simulink model "wlan_phy_rx_pmd"  (Simulink 7.8)
settings: Solver=VariableStepDiscrete  SolverMode=SingleTasking  StartTime=0.0  StopTime=rx_sim.sim_time  RelTol=1e-3
PostLoadFcn:
  | wlan_phy_rx_init
InitFcn:
  | wlan_phy_rx_init
StartFcn:
  | wlan_phy_rx_init
values from neighbouring files (symbols this model uses):
  barker_seq20 = 0.95 * circshift(barker_seq20, [0 4]) ./ max(abs(barker_seq20))    [wlan_phy_rx_init.m]
  bit_scrambler_lfsr_bytes = sum(bit_scrambler_lfsr_bytes .* repmat(2.^[0:7], 127, 1),2)    [wlan_phy_rx_init.m]
  longCorr_coef_i = [3*real(longCorr_coef)]    [wlan_phy_rx_init.m]
  longCorr_coef_q = [3*imag(longCorr_coef)]    [wlan_phy_rx_init.m]
  MAX_CP_LEN = 32    [wlan_phy_rx_init.m]
  MAX_NCBPS = NCBPS_11n_64QAM    (= 312)    [wlan_phy_rx_init.m]
  MAX_NUM_BYTES = 2^16-1    (= 65535)    [wlan_phy_rx_init.m]
  MAX_NUM_SC = 64    [wlan_phy_rx_init.m]
  MAX_NUM_SYMS = 600    [wlan_phy_rx_init.m]
  NDBPS_11a_BPSK12 = 24    [wlan_phy_rx_init.m]
  preFFT_sampBuff_numSamps = 4*MAX_NUM_SC    (= 256)    [wlan_phy_rx_init.m]
  rx_sim = struct()    [wlan_phy_rx_init.m]
  scr_ind_rev = zeros(1,128)    [wlan_phy_rx_init.m]
undefined symbols (not defined in the file or in its folder): gcbh, mcs_rom_11ag, mcs_rom_11n, wlan_rx_deinterleave_rom_gen, xlBlockLoadCallback, xlGetNormalizedPeriod, xlipgetpartsetting
libraries: xbsIndex_r4 x4943, xbsEDKLib_r4 x1
(part 7 of 28)

== system wlan_phy_rx_pmd/Inputs/RF D Inputs ==
  Rst : Inport
  IQ Valid : Inport  Port=2
  Constant1 : Constant  Value=0
  Constant2 : Constant  Value=0
  Constant3 : Constant  Value=0
  Constant6 : Constant  Value=2
  Constant7 : Constant  Value=5
  Delay4 : xbsIndex_r4/Delay  rst=off  infoeditControl=Selection of Reset will increase slice count due to use of real FFs and instead of SRLs  en=off  latency=1  dbl_ovrd=off  reg_retiming=off  block_type=delay
  Delay5 : xbsIndex_r4/Delay  rst=off  infoeditControl=Selection of Reset will increase slice count due to use of real FFs and instead of SRLs  en=off  latency=1  dbl_ovrd=off  reg_retiming=off  block_type=delay
  Down Sample : xbsIndex_r4/Down Sample  sample_ratio=16  sample_phase="Last Value of Frame  (most efficient)"  en=off  latency=1  dbl_ovrd=off  block_type=dsamp
  RF D RSSI Sum : Subsystem
  RFD_G_BB : xbsIndex_r4/Gateway In  gui_display_data_type=Fixed-point  arith_type=Unsigned  n_bits=5  bin_pt=0  preci_type=Single  exp_width=8  frac_width=24  quantization="Round  (unbiased: +/- Inf)"  overflow=Saturate  period=1  dbl_ovrd=off  timing_constraint=None  locs_specified=off  LOCs={}  UseAsADC=off  ADCChannel='1'  inherit_from_input=off  hdl_port=on  block_type=gatewayin
  RFD_G_RF : xbsIndex_r4/Gateway In  gui_display_data_type=Fixed-point  arith_type=Unsigned  n_bits=2  bin_pt=0  preci_type=Single  exp_width=8  frac_width=24  quantization="Round  (unbiased: +/- Inf)"  overflow=Saturate  period=1  dbl_ovrd=off  timing_constraint=None  locs_specified=off  LOCs={}  UseAsADC=off  ADCChannel='1'  inherit_from_input=off  hdl_port=on  block_type=gatewayin
  RFD_RSSI : xbsIndex_r4/Gateway In  gui_display_data_type=Fixed-point  arith_type=Unsigned  n_bits=10  bin_pt=0  preci_type=Single  exp_width=8  frac_width=24  quantization="Round  (unbiased: +/- Inf)"  overflow=Saturate  period=1  dbl_ovrd=off  timing_constraint=None  locs_specified=off  LOCs={}  UseAsADC=off  ADCChannel='1'  inherit_from_input=off  hdl_port=on  block_type=gatewayin
  RFD_RX_I : xbsIndex_r4/Gateway In  gui_display_data_type=Fixed-point  arith_type="Signed  (2's comp)"  n_bits=16  bin_pt=15  preci_type=Single  exp_width=8  frac_width=24  quantization="Round  (unbiased: +/- Inf)"  overflow=Saturate  period=1  dbl_ovrd=off  timing_constraint=None  locs_specified=off  LOCs={}  UseAsADC=off  ADCChannel='1'  inherit_from_input=off  hdl_port=on  block_type=gatewayin
  RFD_RX_Q : xbsIndex_r4/Gateway In  gui_display_data_type=Fixed-point  arith_type="Signed  (2's comp)"  n_bits=16  bin_pt=15  preci_type=Single  exp_width=8  frac_width=24  quantization="Round  (unbiased: +/- Inf)"  overflow=Saturate  period=1  dbl_ovrd=off  timing_constraint=None  locs_specified=off  LOCs={}  UseAsADC=off  ADCChannel='1'  inherit_from_input=off  hdl_port=on  block_type=gatewayin
  Register : xbsIndex_r4/Register  init=0  rst=on  en=on  dbl_ovrd=off  block_type=register
  Register1 : xbsIndex_r4/Register  init=0  rst=on  en=on  dbl_ovrd=off  block_type=register
  Register2 : xbsIndex_r4/Register  init=0  rst=off  en=off  dbl_ovrd=off  block_type=register
  Register3 : xbsIndex_r4/Register  init=0  rst=on  en=off  dbl_ovrd=off  block_type=register
  Register4 : xbsIndex_r4/Register  init=0  rst=off  en=off  dbl_ovrd=off  block_type=register
  Register5 : xbsIndex_r4/Register  init=0  rst=off  en=off  dbl_ovrd=off  block_type=register
  ADC I : Outport
  ADC Q : Outport  Port=2
  Avg RSSI : Outport  Port=3
  tag scope: RFD_AGC_G_BB=global, RFD_AGC_G_RF=global
  wires:
  Constant7/1 -> RFD_G_BB/1
  Constant6/1 -> RFD_G_RF/1
  Delay5/1 -> [RFD_AGC_G_BB]
  Delay4/1 -> [RFD_AGC_G_RF]
  RFD_G_BB/1 -> Delay5/1
  RFD_G_RF/1 -> Delay4/1
  RFD_RX_I/1 -> Register2/1
  RFD_RX_Q/1 -> Register4/1
  Register/1 -> ADC I/1
  Register1/1 -> ADC Q/1
  Register3/1 -> Down Sample/1
  Down Sample/1 -> RF D RSSI Sum/1(RSSI)
  RF D RSSI Sum/1(Sum) -> Avg RSSI/1
  RFD_RSSI/1 -> Register5/1
  Register2/1 -> Register/1
  Register4/1 -> Register1/1
  Register5/1 -> Register3/1
  Rst/1 -> Register/2, Register1/2, Register3/2
  IQ Valid/1 -> Register1/3, Register/3
  Constant1/1 -> RFD_RX_I/1
  Constant2/1 -> RFD_RX_Q/1
  Constant3/1 -> RFD_RSSI/1

== system wlan_phy_rx_pmd/Inputs/RSSI Clock Gen ==
  1LSB+3 : xbsIndex_r4/Slice  nbits=1  boolean_output=off  mode=Lower Bit Location + Width  bit1=0  base1=MSB of Input  bit0=3  base0=LSB of Input  dbl_ovrd=off  block_type=slice
  RSSI Clock Generator : xbsIndex_r4/Counter  cnt_type=Free Running  cnt_to=Inf  operation=Up  start_count=0  cnt_by_val=1  arith_type=Unsigned  n_bits=4  bin_pt=0  load_pin=off  rst=off  en=off  explicit_period=on  period=1  dbl_ovrd=off  use_behavioral_HDL=off  implementation=Fabric  use_rpm=off  block_type=counter
      settings: block_version=VER_STRING_GOES_HERE
  Clk : Outport
  wires:
  RSSI Clock Generator/1 -> 1LSB+3/1
  1LSB+3/1 -> Clk/1

== system wlan_phy_rx_pmd/Inputs/negedge1 ==
  D : Inport
  Delay : xbsIndex_r4/Delay  rst=off  infoeditControl=Selection of Reset will increase slice count due to use of real FFs and instead of SRLs  en=off  latency=1  dbl_ovrd=off  reg_retiming=off  block_type=delay
      settings: infoedit=Hardware notes: A delay line is a chain, each link of which is an SRL16 followed by a flip-flop. If register retiming is enabled, the delay line is a chain of flip-flops.  xl_area=[1 1 0 0 0 0 0]  block_version=VER_STRING_GOES_HERE
  Inverter : xbsIndex_r4/Inverter  en=off  latency=0  dbl_ovrd=off  block_type=inv
      settings: xl_area=[0 0 0 0 0 0 0]  block_version=VER_STRING_GOES_HERE
  Logical1 : xbsIndex_r4/Logical  logical_function=AND  inputs=2  en=off  latency=0  precision=Full  arith_type=Unsigned  n_bits=8  bin_pt=2  align_bp=on  dbl_ovrd=off  block_type=logical
      settings: xl_area=[1 0 0 1 0 0 0]  block_version=VER_STRING_GOES_HERE
  Q : Outport
  wires:
  D/1 -> Delay/1, Inverter/1
  Logical1/1 -> Q/1
  Delay/1 -> Logical1/1
  Inverter/1 -> Logical1/2

== system wlan_phy_rx_pmd/MAC I/O/Pulse Stretch1 ==
  P : Inport
  Delay : xbsIndex_r4/Delay  rst=off  infoeditControl=Selection of Reset will increase slice count due to use of real FFs and instead of SRLs  en=off  latency=16  dbl_ovrd=off  reg_retiming=off  block_type=delay
  Register15 : xbsIndex_r4/Register  init=0  rst=off  en=off  dbl_ovrd=off  block_type=register
  S-R Latch : Subsystem
  P16 : Outport
  wires:
  S-R Latch/1(Q) -> Delay/1, Register15/1
  Delay/1 -> S-R Latch/2(R)
  P/1 -> S-R Latch/1(S)
  Register15/1 -> P16/1

== system wlan_phy_rx_pmd/MAC I/O/S-R Latch1 ==
  S : Inport
  R : Inport  Port=2
  Constant1 : xbsIndex_r4/Constant  const=1  gui_display_data_type=Fixed-point  arith_type=Boolean  n_bits=1  bin_pt=0  preci_type=Single  exp_width=8  frac_width=24  explicit_period=off  period=1  equ=P=C  opselect=C  inp2=PCIN>>17  opr=+  inp1=P  carry=CIN  dbl_ovrd=off  block_type=constant
      settings: block_version=VER_STRING_GOES_HERE
  Convert1 : xbsIndex_r4/Convert  gui_display_data_type=Boolean  arith_type=Boolean  n_bits=1  bin_pt=0  float_type=Single  exp_bits=8  fraction_bits=24  quantization=Truncate  overflow=Wrap  en=off  latency=0  dbl_ovrd=off  pipeline=off  block_type=convert
  Convert2 : xbsIndex_r4/Convert  gui_display_data_type=Boolean  arith_type=Boolean  n_bits=1  bin_pt=0  float_type=Single  exp_bits=8  fraction_bits=24  quantization=Truncate  overflow=Wrap  en=off  latency=0  dbl_ovrd=off  pipeline=off  block_type=convert
  Register2 : xbsIndex_r4/Register  init=0  rst=on  en=on  dbl_ovrd=off  block_type=register
      settings: xl_area=[1 1 0 0 0 0 0]  block_version=VER_STRING_GOES_HERE
  Q : Outport
  wires:
  Convert2/1 -> Register2/3
  Convert1/1 -> Register2/2
  Constant1/1 -> Register2/1
  R/1 -> Convert1/1
  Register2/1 -> Q/1
  S/1 -> Convert2/1

== system wlan_phy_rx_pmd/MAC I/O/S-R Latch2 ==
  S : Inport
  R : Inport  Port=2
  Constant1 : xbsIndex_r4/Constant  const=1  gui_display_data_type=Fixed-point  arith_type=Boolean  n_bits=1  bin_pt=0  preci_type=Single  exp_width=8  frac_width=24  explicit_period=off  period=1  equ=P=C  opselect=C  inp2=PCIN>>17  opr=+  inp1=P  carry=CIN  dbl_ovrd=off  block_type=constant
      settings: block_version=VER_STRING_GOES_HERE
  Convert1 : xbsIndex_r4/Convert  gui_display_data_type=Boolean  arith_type=Boolean  n_bits=1  bin_pt=0  float_type=Single  exp_bits=8  fraction_bits=24  quantization=Truncate  overflow=Wrap  en=off  latency=0  dbl_ovrd=off  pipeline=off  block_type=convert
  Convert2 : xbsIndex_r4/Convert  gui_display_data_type=Boolean  arith_type=Boolean  n_bits=1  bin_pt=0  float_type=Single  exp_bits=8  fraction_bits=24  quantization=Truncate  overflow=Wrap  en=off  latency=0  dbl_ovrd=off  pipeline=off  block_type=convert
  Register2 : xbsIndex_r4/Register  init=0  rst=on  en=on  dbl_ovrd=off  block_type=register
      settings: xl_area=[1 1 0 0 0 0 0]  block_version=VER_STRING_GOES_HERE
  Q : Outport
  wires:
  S/1 -> Convert2/1
  Register2/1 -> Q/1
  R/1 -> Convert1/1
  Constant1/1 -> Register2/1
  Convert1/1 -> Register2/2
  Convert2/1 -> Register2/3

== system wlan_phy_rx_pmd/MAC I/O/posedge ==
  D : Inport
  Delay : xbsIndex_r4/Delay  rst=off  infoeditControl=Selection of Reset will increase slice count due to use of real FFs and instead of SRLs  en=off  latency=1  dbl_ovrd=off  reg_retiming=off  block_type=delay
      settings: infoedit=Hardware notes: A delay line is a chain, each link of which is an SRL16 followed by a flip-flop. If register retiming is enabled, the delay line is a chain of flip-flops.  xl_area=[1 1 0 0 0 0 0]  block_version=VER_STRING_GOES_HERE
  Inverter : xbsIndex_r4/Inverter  en=off  latency=0  dbl_ovrd=off  block_type=inv
      settings: xl_area=[0 0 0 0 0 0 0]  block_version=VER_STRING_GOES_HERE
  Logical1 : xbsIndex_r4/Logical  logical_function=AND  inputs=2  en=off  latency=0  precision=Full  arith_type=Unsigned  n_bits=8  bin_pt=2  align_bp=on  dbl_ovrd=off  block_type=logical
      settings: xl_area=[1 0 0 1 0 0 0]  block_version=VER_STRING_GOES_HERE
  Q : Outport
  wires:
  Inverter/1 -> Logical1/2
  Logical1/1 -> Q/1
  D/1 -> Delay/1, Logical1/1
  Delay/1 -> Inverter/1

== system wlan_phy_rx_pmd/Registers/2x  ==
  d : Inport
  Concat2 : xbsIndex_r4/Concat  num_inputs=2  dbl_ovrd=off  block_type=concat
      settings: block_version=8.2.02
  Constant2 : xbsIndex_r4/Constant  const=0  gui_display_data_type=Fixed-point  arith_type=Unsigned  n_bits=1  bin_pt=0  preci_type=Single  exp_width=8  frac_width=24  explicit_period=on  period=1  equ=P=C  opselect=C  inp2=PCIN>>17  opr=+  inp1=P  carry=CIN  dbl_ovrd=off  block_type=constant
  2d : Outport
  wires:
  Concat2/1 -> 2d/1
  d/1 -> Concat2/1
  Constant2/1 -> Concat2/2

== system wlan_phy_rx_pmd/Registers/Config Bits ==
  32b : Inport
  "b[0]   " : xbsIndex_r4/Slice  nbits=1  boolean_output=on  mode=Lower Bit Location + Width  bit1=0  base1=MSB of Input  bit0=0  base0=LSB of Input  dbl_ovrd=off  block_type=slice
      settings: block_version=VER_STRING_GOES_HERE
  b[10] : xbsIndex_r4/Slice  nbits=1  boolean_output=on  mode=Lower Bit Location + Width  bit1=0  base1=MSB of Input  bit0=10  base0=LSB of Input  dbl_ovrd=off  block_type=slice
      settings: block_version=VER_STRING_GOES_HERE
  b[11] : xbsIndex_r4/Slice  nbits=1  boolean_output=on  mode=Lower Bit Location + Width  bit1=0  base1=MSB of Input  bit0=11  base0=LSB of Input  dbl_ovrd=off  block_type=slice
      settings: block_version=VER_STRING_GOES_HERE
  b[12] : xbsIndex_r4/Slice  nbits=1  boolean_output=on  mode=Lower Bit Location + Width  bit1=0  base1=MSB of Input  bit0=12  base0=LSB of Input  dbl_ovrd=off  block_type=slice
      settings: block_version=VER_STRING_GOES_HERE
  b[13] : xbsIndex_r4/Slice  nbits=1  boolean_output=on  mode=Lower Bit Location + Width  bit1=0  base1=MSB of Input  bit0=13  base0=LSB of Input  dbl_ovrd=off  block_type=slice
      settings: block_version=VER_STRING_GOES_HERE
  b[14] : xbsIndex_r4/Slice  nbits=1  boolean_output=on  mode=Lower Bit Location + Width  bit1=0  base1=MSB of Input  bit0=14  base0=LSB of Input  dbl_ovrd=off  block_type=slice
      settings: block_version=VER_STRING_GOES_HERE
  b[16:15] : xbsIndex_r4/Slice  nbits=2  boolean_output=off  mode=Lower Bit Location + Width  bit1=0  base1=MSB of Input  bit0=15  base0=LSB of Input  dbl_ovrd=off  block_type=slice
      settings: block_version=VER_STRING_GOES_HERE
  "b[1]  " : xbsIndex_r4/Slice  nbits=1  boolean_output=on  mode=Lower Bit Location + Width  bit1=0  base1=MSB of Input  bit0=1  base0=LSB of Input  dbl_ovrd=off  block_type=slice
      settings: block_version=VER_STRING_GOES_HERE
  b[20:17] : xbsIndex_r4/Slice  nbits=4  boolean_output=off  mode=Lower Bit Location + Width  bit1=0  base1=MSB of Input  bit0=17  base0=LSB of Input  dbl_ovrd=off  block_type=slice
      settings: block_version=VER_STRING_GOES_HERE
  b[21] : xbsIndex_r4/Slice  nbits=1  boolean_output=on  mode=Lower Bit Location + Width  bit1=0  base1=MSB of Input  bit0=21  base0=LSB of Input  dbl_ovrd=off  block_type=slice
      settings: block_version=VER_STRING_GOES_HERE
  b[22] : xbsIndex_r4/Slice  nbits=1  boolean_output=on  mode=Lower Bit Location + Width  bit1=0  base1=MSB of Input  bit0=22  base0=LSB of Input  dbl_ovrd=off  block_type=slice
      settings: block_version=VER_STRING_GOES_HERE
  b[23] : xbsIndex_r4/Slice  nbits=1  boolean_output=on  mode=Lower Bit Location + Width  bit1=0  base1=MSB of Input  bit0=23  base0=LSB of Input  dbl_ovrd=off  block_type=slice
      settings: block_version=VER_STRING_GOES_HERE
  b[24] : xbsIndex_r4/Slice  nbits=1  boolean_output=on  mode=Lower Bit Location + Width  bit1=0  base1=MSB of Input  bit0=24  base0=LSB of Input  dbl_ovrd=off  block_type=slice
      settings: block_version=VER_STRING_GOES_HERE
  b[2] : xbsIndex_r4/Slice  nbits=1  boolean_output=off  mode=Lower Bit Location + Width  bit1=0  base1=MSB of Input  bit0=2  base0=LSB of Input  dbl_ovrd=off  block_type=slice
      settings: block_version=VER_STRING_GOES_HERE
  b[3] : xbsIndex_r4/Slice  nbits=1  boolean_output=off  mode=Lower Bit Location + Width  bit1=0  base1=MSB of Input  bit0=3  base0=LSB of Input  dbl_ovrd=off  block_type=slice
      settings: block_version=VER_STRING_GOES_HERE
  b[4] : xbsIndex_r4/Slice  nbits=1  boolean_output=off  mode=Lower Bit Location + Width  bit1=0  base1=MSB of Input  bit0=4  base0=LSB of Input  dbl_ovrd=off  block_type=slice
      settings: block_version=VER_STRING_GOES_HERE
  b[5] : xbsIndex_r4/Slice  nbits=1  boolean_output=on  mode=Lower Bit Location + Width  bit1=0  base1=MSB of Input  bit0=5  base0=LSB of Input  dbl_ovrd=off  block_type=slice
      settings: block_version=VER_STRING_GOES_HERE
  b[6] : xbsIndex_r4/Slice  nbits=1  boolean_output=on  mode=Lower Bit Location + Width  bit1=0  base1=MSB of Input  bit0=6  base0=LSB of Input  dbl_ovrd=off  block_type=slice
      settings: block_version=VER_STRING_GOES_HERE
  b[7] : xbsIndex_r4/Slice  nbits=1  boolean_output=on  mode=Lower Bit Location + Width  bit1=0  base1=MSB of Input  bit0=7  base0=LSB of Input  dbl_ovrd=off  block_type=slice
      settings: block_version=VER_STRING_GOES_HERE
  b[8] : xbsIndex_r4/Slice  nbits=1  boolean_output=on  mode=Lower Bit Location + Width  bit1=0  base1=MSB of Input  bit0=8  base0=LSB of Input  dbl_ovrd=off  block_type=slice
      settings: block_version=VER_STRING_GOES_HERE
  b[9] : xbsIndex_r4/Slice  nbits=1  boolean_output=on  mode=Lower Bit Location + Width  bit1=0  base1=MSB of Input  bit0=9  base0=LSB of Input  dbl_ovrd=off  block_type=slice
      settings: block_version=VER_STRING_GOES_HERE
  tag scope: REG_USE_RX_SIGS_INVALID_INPUT=global, regRx_BYPASS_CFO_EST=global, regRx_DSSS_RX_EN=global, regRx_DSSS_RX_PKT_DET_OUT_EN=global, regRx_DSSS_RX_REQ_AGC_DONE=global, regRx_DSSS_asserts_CCA_busy=global, regRx_MAX_SIGNAL_LENGTH_KB=global, regRx_PHY_MODE_11N_ENABLE=global, regRx_PKT_BUF_BYTE_ORDER_SWAP=global, regRx_PKT_BUF_H_EST_ORDER_SWAP=global, regRx_Record_Chan_Est_Pkt_Buf=global, regRx_noSwitching_AntSel=global, regRx_phyCCA_Mode_Sel=global, regRx_pktDetEn_Ext=global, regRx_pktDetEn_RFA=global, regRx_pktDetEn_RFB=global, regRx_pktDetEn_RFC=global, regRx_pktDetEn_RFD=global, regRx_pktDet_requireBoth=global, regRx_rateLength_holds_pktDet=global, regRx_switchingDiv_En=global
  wires:
  32b/1 -> "b[1]  "/1, b[2]/1, b[3]/1, b[4]/1, b[5]/1, b[6]/1, b[7]/1, b[9]/1, b[10]/1, b[12]/1, b[13]/1, b[14]/1, b[16:15]/1, b[20:17]/1, b[21]/1, b[22]/1, b[23]/1, b[24]/1, b[11]/1, b[8]/1, "b[0]   "/1
  "b[0]   "/1 -> [regRx_DSSS_RX_EN]
  "b[1]  "/1 -> [REG_USE_RX_SIGS_INVALID_INPUT]
  b[2]/1 -> [regRx_PKT_BUF_BYTE_ORDER_SWAP]
  b[3]/1 -> [regRx_PKT_BUF_H_EST_ORDER_SWAP]
  b[4]/1 -> [regRx_DSSS_RX_PKT_DET_OUT_EN]
  b[5]/1 -> [regRx_BYPASS_CFO_EST]
  b[6]/1 -> [regRx_Record_Chan_Est_Pkt_Buf]
  b[7]/1 -> [regRx_switchingDiv_En]
  b[16:15]/1 -> [regRx_noSwitching_AntSel]
  b[9]/1 -> [regRx_pktDetEn_RFA]
  b[10]/1 -> [regRx_pktDetEn_RFB]
  b[13]/1 -> [regRx_pktDetEn_Ext]
  b[11]/1 -> [regRx_pktDetEn_RFC]
  b[12]/1 -> [regRx_pktDetEn_RFD]
  b[14]/1 -> [regRx_phyCCA_Mode_Sel]
  b[8]/1 -> [regRx_DSSS_RX_REQ_AGC_DONE]
  b[20:17]/1 -> [regRx_MAX_SIGNAL_LENGTH_KB]
  b[21]/1 -> [regRx_pktDet_requireBoth]
  b[22]/1 -> [regRx_rateLength_holds_pktDet]
  b[23]/1 -> [regRx_DSSS_asserts_CCA_busy]
  b[24]/1 -> [regRx_PHY_MODE_11N_ENABLE]

== system wlan_phy_rx_pmd/Registers/Fanout ==
  Corr_Thresh : Inport
  E_Thresh : Inport  Port=2
  MinDur : Inport  Port=3
  Register{1..11,20} (12 alike) : xbsIndex_r4/Register  init=0  rst=off  en=off  dbl_ovrd=off  block_type=register
  tag scope: regPktDet_pktDetMinDuration_autoCorr_A=global, regPktDet_pktDetMinDuration_autoCorr_B=global, regPktDet_pktDetMinDuration_autoCorr_C=global, regPktDet_pktDetMinDuration_autoCorr_D=global, regRx_PktDet_CorrThresh_A=global, regRx_PktDet_CorrThresh_B=global, regRx_PktDet_CorrThresh_C=global, regRx_PktDet_CorrThresh_D=global, regRx_PktDet_EnergyThresh_A=global, regRx_PktDet_EnergyThresh_B=global, regRx_PktDet_EnergyThresh_C=global, regRx_PktDet_EnergyThresh_D=global
  wires:
  Corr_Thresh/1 -> Register20/1, Register1/1, Register2/1, Register3/1
  E_Thresh/1 -> Register4/1, Register5/1, Register6/1, Register7/1
  MinDur/1 -> Register8/1, Register9/1, Register10/1, Register11/1
  Register20/1 -> [regRx_PktDet_CorrThresh_D]
  Register1/1 -> [regRx_PktDet_CorrThresh_C]
  Register2/1 -> [regRx_PktDet_CorrThresh_B]
  Register3/1 -> [regRx_PktDet_CorrThresh_A]
  Register4/1 -> [regRx_PktDet_EnergyThresh_A]
  Register5/1 -> [regRx_PktDet_EnergyThresh_B]
  Register6/1 -> [regRx_PktDet_EnergyThresh_C]
  Register7/1 -> [regRx_PktDet_EnergyThresh_D]
  Register8/1 -> [regPktDet_pktDetMinDuration_autoCorr_A]
  Register9/1 -> [regPktDet_pktDetMinDuration_autoCorr_B]
  Register10/1 -> [regPktDet_pktDetMinDuration_autoCorr_C]
  Register11/1 -> [regPktDet_pktDetMinDuration_autoCorr_D]

== system wlan_phy_rx_pmd/Registers/Fanout1 ==
  In1 : Inport
  In2 : Inport  Port=2
  In3 : Inport  Port=3
  Register{1..11,20} (12 alike) : xbsIndex_r4/Register  init=0  rst=off  en=off  dbl_ovrd=off  block_type=register
  tag scope: regRx_PKTDET_RSSI_MIN_DUR_A=global, regRx_PKTDET_RSSI_MIN_DUR_B=global, regRx_PKTDET_RSSI_MIN_DUR_C=global, regRx_PKTDET_RSSI_MIN_DUR_D=global, regRx_PKTDET_RSSI_THRESH_A=global, regRx_PKTDET_RSSI_THRESH_B=global, regRx_PKTDET_RSSI_THRESH_C=global, regRx_PKTDET_RSSI_THRESH_D=global, regRx_RSSI_SUM_LEN_A=global, regRx_RSSI_SUM_LEN_B=global, regRx_RSSI_SUM_LEN_C=global, regRx_RSSI_SUM_LEN_D=global
  wires:
  Register3/1 -> [regRx_RSSI_SUM_LEN_A]
  Register2/1 -> [regRx_RSSI_SUM_LEN_B]
  Register1/1 -> [regRx_RSSI_SUM_LEN_C]
  Register20/1 -> [regRx_RSSI_SUM_LEN_D]
  In1/1 -> Register3/1, Register2/1, Register1/1, Register20/1
  In2/1 -> Register6/1, Register4/1, Register5/1, Register7/1
  In3/1 -> Register11/1, Register9/1, Register8/1, Register10/1
  Register11/1 -> [regRx_PKTDET_RSSI_MIN_DUR_A]
  Register9/1 -> [regRx_PKTDET_RSSI_MIN_DUR_B]
  Register8/1 -> [regRx_PKTDET_RSSI_MIN_DUR_C]
  Register10/1 -> [regRx_PKTDET_RSSI_MIN_DUR_D]
  Register7/1 -> [regRx_PKTDET_RSSI_THRESH_A]
  Register5/1 -> [regRx_PKTDET_RSSI_THRESH_B]
  Register4/1 -> [regRx_PKTDET_RSSI_THRESH_C]
  Register6/1 -> [regRx_PKTDET_RSSI_THRESH_D]

== system wlan_phy_rx_pmd/Registers/Fanout2 ==
  Corr_Thresh : Inport
  E_Thresh : Inport  Port=2
  Register{1..7,20} (8 alike) : xbsIndex_r4/Register  init=0  rst=off  en=off  dbl_ovrd=off  block_type=register
  tag scope: regRx_PktDet_CorrThresh_DSSS_A=global, regRx_PktDet_CorrThresh_DSSS_B=global, regRx_PktDet_CorrThresh_DSSS_C=global, regRx_PktDet_CorrThresh_DSSS_D=global, regRx_PktDet_EnergyThresh_DSSS_A=global, regRx_PktDet_EnergyThresh_DSSS_B=global, regRx_PktDet_EnergyThresh_DSSS_C=global, regRx_PktDet_EnergyThresh_DSSS_D=global
  wires:
  Register3/1 -> [regRx_PktDet_CorrThresh_DSSS_A]
  Register2/1 -> [regRx_PktDet_CorrThresh_DSSS_B]
  Register1/1 -> [regRx_PktDet_CorrThresh_DSSS_C]
  Register20/1 -> [regRx_PktDet_CorrThresh_DSSS_D]
  Corr_Thresh/1 -> Register3/1, Register2/1, Register1/1, Register20/1
  E_Thresh/1 -> Register4/1, Register5/1, Register6/1, Register7/1
  Register4/1 -> [regRx_PktDet_EnergyThresh_DSSS_A]
  Register5/1 -> [regRx_PktDet_EnergyThresh_DSSS_B]
  Register6/1 -> [regRx_PktDet_EnergyThresh_DSSS_C]
  Register7/1 -> [regRx_PktDet_EnergyThresh_DSSS_D]

== system wlan_phy_rx_pmd/Registers/Sim Reset ==
  Add : Sum
  Disregard Subsystem : xbsIndex_r4/Disregard Subsystem  Tag=discardX  block_type=disregard
  Pulse Generator : DiscretePulseGenerator  Period=1e6  PulseWidth=2  PhaseDelay=1.25e4
  Pulse Generator1 : DiscretePulseGenerator  Period=1e6  PulseWidth=2  PhaseDelay=2.5e4
  sim_only_reset : xbsIndex_r4/Gateway In  gui_display_data_type=Fixed-point  arith_type=Unsigned  n_bits=1  bin_pt=0  preci_type=Single  exp_width=8  frac_width=24  quantization="Round  (unbiased: +/- Inf)"  overflow=Saturate  period=1  dbl_ovrd=off  timing_constraint=None  locs_specified=off  LOCs={}  UseAsADC=off  ADCChannel='1'  inherit_from_input=off  hdl_port=on  block_type=gatewayin
  Out1 : Outport
  wires:
  sim_only_reset/1 -> Out1/1
  Pulse Generator/1 -> Add/1
  Pulse Generator1/1 -> Add/2
  Add/1 -> sim_only_reset/1

== system wlan_phy_rx_pmd/Sync & Antenna Sel/Antenna Selection ==
  Pkt Det : Inport
  Rx I Vec : Inport  Port=2
  Rx Q Vec : Inport  Port=3
  IQ Valid : Inport  Port=4
  RSSI Vec : Inport  Port=5
  Reset : Inport  Port=6
  Ant Sel Logic : Subsystem
  Bus Selector1 : BusSelector  OutputSignals=RFA_Q,RFB_Q,RFC_Q,RFD_Q
  Bus Selector2 : BusSelector  OutputSignals=RFA_RSSI,RFB_RSSI,RFC_RSSI,RFD_RSSI
  Bus Selector3 : BusSelector  OutputSignals=RFA_I,RFB_I,RFC_I,RFD_I
  Bus Selector4 : BusSelector  OutputSignals=signal1,signal2,signal3,signal4
  Delay : xbsIndex_r4/Delay  rst=off  infoeditControl=Selection of Reset will increase slice count due to use of real FFs and instead of SRLs  en=off  latency=1  dbl_ovrd=off  reg_retiming=off  block_type=delay
  Gateway Out{,1..2,4..6} (6 alike) : xbsIndex_r4/Gateway Out  inherit_from_input=off  hdl_port=off  timing_constraint=None  locs_specified=off  LOCs={}  UseAsDAC=off  DACChannel='1'  block_type=gatewayout
  Logical : xbsIndex_r4/Logical  logical_function=AND  inputs=2  en=off  latency=0  precision=Full  arith_type=Unsigned  n_bits=16  bin_pt=0  align_bp=on  dbl_ovrd=off  block_type=logical
  Mux{1..4} (4 alike) : xbsIndex_r4/Mux  inputs=4  en=off  latency=0  precision=Full  arith_type=Unsigned  n_bits=16  bin_pt=14  quantization=Truncate  overflow=Wrap  dbl_ovrd=off  block_type=mux
  Posedge : Subsystem
  RF A Gains : Subsystem
  RF B Gains : Subsystem
  RF C Gains : Subsystem
  RF D Gains : Subsystem
  RSSI & Gain Capture : Subsystem
  Register3 : xbsIndex_r4/Register  init=0  rst=off  en=on  dbl_ovrd=off  block_type=register
      settings: xl_area=[1 1 0 0 0 0 0]  block_version=VER_STRING_GOES_HERE
  Sel Div : Scope  NumInputPorts=10
      settings: TimeRange=16708  YMin=0~-1~-1~0~-1~-1~-1~-1~-1~-1  YMax=1~1~1~1~1~1~1~1~1~1  SaveName=ScopeData42
  Rx I : Outport
  " Rx Q" : Outport  Port=2
  " IQ Valid" : Outport  Port=3
  RSSI : Outport  Port=4
  tag scope: ANTENNA_SEL=global
  wires:
  RF A Gains/1(G_vec) -> Gateway Out2/1, Ant Sel Logic/1(G A)
  RF B Gains/1(G_vec) -> Gateway Out4/1, Ant Sel Logic/2(G B)
  Rx Q Vec/1 -> Bus Selector1/1
  Reset/1 -> RF B Gains/2(Rst), RF A Gains/2(Rst), RF C Gains/2(Rst), RF D Gains/2(Rst)
  Logical/1 -> Posedge/1(D)
  Pkt Det/1 -> Logical/1, Gateway Out/1
  [AGC_DONE] -> Logical/2, Gateway Out1/1
  Posedge/1(Q) -> RF A Gains/1(Capt), RF B Gains/1(Capt), RF C Gains/1(Capt), Delay/1, RF D Gains/1(Capt)
  Delay/1 -> Register3/2
  Register3/1 -> [ANTENNA_SEL]
  IQ Valid/1 -> Bus Selector4/1
  Bus Selector4/1 -> Mux3/2  "<signal1>"
  Bus Selector4/2 -> Mux3/3  "<signal2>"
  Mux3/1 -> " IQ Valid"/1
  Gateway Out2/1 -> Sel Div/3  "G A"
  Gateway Out1/1 -> Sel Div/2  "AGC Done"
  Gateway Out/1 -> Sel Div/1  "Pkt Det"
  Gateway Out4/1 -> Sel Div/4  "G B"
  RF C Gains/1(G_vec) -> Ant Sel Logic/3(G C), Gateway Out5/1
  RF D Gains/1(G_vec) -> Ant Sel Logic/4(G D), Gateway Out6/1
  Gateway Out5/1 -> Sel Div/5  "G C"
  Gateway Out6/1 -> Sel Div/6  "G D"
  Bus Selector4/3 -> Mux3/4  "<signal3>"
  Bus Selector4/4 -> Mux3/5  "<signal4>"
  Rx I Vec/1 -> Bus Selector3/1
  Bus Selector3/1 -> Mux1/2  "<RFA_I>"
  Bus Selector3/2 -> Mux1/3  "<RFB_I>"
  Bus Selector3/3 -> Mux1/4  "<RFC_I>"
  Bus Selector3/4 -> Mux1/5  "<RFD_I>"
  Bus Selector1/1 -> Mux2/2  "<RFA_Q>"
  Bus Selector1/2 -> Mux2/3  "<RFB_Q>"
  Bus Selector1/3 -> Mux2/4  "<RFC_Q>"
  Bus Selector1/4 -> Mux2/5  "<RFD_Q>"
  RSSI Vec/1 -> Bus Selector2/1, RSSI & Gain Capture/1(RSSI Vec)
  Bus Selector2/1 -> Mux4/2  "<RFA_RSSI>"
  Bus Selector2/2 -> Mux4/3  "<RFB_RSSI>"
  Bus Selector2/3 -> Mux4/4  "<RFC_RSSI>"
  Bus Selector2/4 -> Mux4/5  "<RFD_RSSI>"
  Mux4/1 -> RSSI/1
  Ant Sel Logic/1(Ant Sel) -> Mux3/1, Mux1/1, Mux2/1, Mux4/1, Register3/1
  Mux2/1 -> " Rx Q"/1
  Mux1/1 -> Rx I/1

== system wlan_phy_rx_pmd/Sync & Antenna Sel/Debug  Outputs ==
  note: Pulse stretch the timeout signal
      | so it's visible on debug outputs
      | and in down-sampled CS ILA
  LTS : Inport
  LTS Timeout : Inport  Port=2
  Det OFDM : Inport  Port=3
  Det DSSS : Inport  Port=4
  LTS_SYNC : xbsIndex_r4/Gateway Out  inherit_from_input=off  hdl_port=on  timing_constraint=None  locs_specified=off  LOCs={}  UseAsDAC=off  DACChannel='1'  block_type=gatewayout
  Pulse Stretch : Subsystem
  Pulse Stretch1 : Subsystem
  Register{2..11} (10 alike) : xbsIndex_r4/Register  init=0  rst=off  en=off  dbl_ovrd=off  block_type=register
  Slice2 : xbsIndex_r4/Slice  nbits=1  boolean_output=on  mode=Upper Bit Location + Width  bit1=0  base1=MSB of Input  bit0=0  base0=LSB of Input  dbl_ovrd=off  block_type=slice
  dbg_PKT_DET_DSSS : xbsIndex_r4/Gateway Out  inherit_from_input=off  hdl_port=on  timing_constraint=None  locs_specified=off  LOCs={}  UseAsDAC=off  DACChannel='1'  block_type=gatewayout
  dbg_PKT_DET_OFDM : xbsIndex_r4/Gateway Out  inherit_from_input=off  hdl_port=on  timing_constraint=None  locs_specified=off  LOCs={}  UseAsDAC=off  DACChannel='1'  block_type=gatewayout
  dbg_lts_timeout : xbsIndex_r4/Gateway Out  inherit_from_input=off  hdl_port=on  timing_constraint=None  locs_specified=off  LOCs={}  UseAsDAC=off  DACChannel='1'  block_type=gatewayout
  tag scope: CS_LTS_Timeout=global, CS_Pktdet_DSSS=global, CS_Pktdet_OFDM=global
  wires:
  Register8/1 -> Register9/1
  Register9/1 -> LTS_SYNC/1
  Slice2/1 -> Pulse Stretch/1(P)
  Register2/1 -> Register3/1
  Register3/1 -> dbg_lts_timeout/1
  LTS/1 -> Slice2/1
  Register6/1 -> Register4/1, [CS_Pktdet_OFDM]
  Register7/1 -> dbg_PKT_DET_OFDM/1
  Register4/1 -> Register7/1
  Register10/1 -> Register5/1, [CS_Pktdet_DSSS]
  Register11/1 -> dbg_PKT_DET_DSSS/1
  Register5/1 -> Register11/1
  Det OFDM/1 -> Register6/1
  Det DSSS/1 -> Register10/1
  Pulse Stretch/1(P16) -> Register8/1
  LTS Timeout/1 -> Pulse Stretch1/1(P)
  Pulse Stretch1/1(P16) -> Register2/1, [CS_LTS_Timeout]

== system wlan_phy_rx_pmd/Sync & Antenna Sel/LTS Correlation ==
  rx_I : Inport
  rx_Q : Inport  Port=2
  rx_IQ_valid : Inport  Port=3
  RSSI : Inport  Port=4
  En : Inport  Port=5
  Reset : Inport  Port=6
  Bus Creator : BusCreator  Inputs=2
  Bus Creator1 : BusCreator  Inputs=2
  Concat : xbsIndex_r4/Concat  num_inputs=2  dbl_ovrd=off  block_type=concat
  Correlator : Subsystem
  Gateway Out{1..10} (10 alike) : xbsIndex_r4/Gateway Out  inherit_from_input=off  hdl_port=off  timing_constraint=None  locs_specified=off  LOCs={}  UseAsDAC=off  DACChannel='1'  block_type=gatewayout
  Inverter : xbsIndex_r4/Inverter  en=off  latency=0  dbl_ovrd=off  block_type=inv
      settings: xl_area=[0 0 0 0 0 0 0]  block_version=VER_STRING_GOES_HERE
  Inverter1 : xbsIndex_r4/Inverter  en=off  latency=0  dbl_ovrd=off  block_type=inv
      settings: xl_area=[0 0 0 0 0 0 0]  block_version=VER_STRING_GOES_HERE
  LTS Corr : Scope  NumInputPorts=8
      settings: TimeRange=30000  YMin=0~-0.5~-0.5~0~0~0~0~-1  YMax=1~0.6~0.5~25000~1~1~1~1  SaveName=ScopeData2
  LTS Corr Timeout : Subsystem
  Logical1 : xbsIndex_r4/Logical  logical_function=AND  inputs=2  en=off  latency=0  precision=Full  arith_type=Unsigned  n_bits=16  bin_pt=0  align_bp=on  dbl_ovrd=off  block_type=logical
  Logical2 : xbsIndex_r4/Logical  logical_function=AND  inputs=3  en=off  latency=0  precision=Full  arith_type=Unsigned  n_bits=16  bin_pt=0  align_bp=on  dbl_ovrd=off  block_type=logical
  Logical3 : xbsIndex_r4/Logical  logical_function=AND  inputs=2  en=off  latency=1  precision=Full  arith_type=Unsigned  n_bits=16  bin_pt=0  align_bp=on  dbl_ovrd=off  block_type=logical
  Posedge3 : Subsystem
  Relational2 : xbsIndex_r4/Relational  mode=a>b  en=off  latency=0  dbl_ovrd=off  block_type=relational
      settings: xl_area=[10 0 0 19 0 0 0]  block_version=VER_STRING_GOES_HERE
  S-R Latch : Subsystem
  S-R Latch1 : Subsystem
  Slice : xbsIndex_r4/Slice  nbits=1  boolean_output=off  mode=Upper Bit Location + Width  bit1=0  base1=MSB of Input  bit0=0  base0=LSB of Input  dbl_ovrd=off  block_type=slice
      settings: infoedit="Extracts a given range of bits from each input sample and presents it at the output.  The output type is ordinarily unsigned with binary point at zero, but can be Boolean when the slice is one bit wide.<P><P>Hardware notes: In hardware this block costs nothing."  block_version=10.1.3
  Slice1 : xbsIndex_r4/Slice  nbits=1  boolean_output=off  mode=Upper Bit Location + Width  bit1=0  base1=MSB of Input  bit0=0  base0=LSB of Input  dbl_ovrd=off  block_type=slice
      settings: infoedit="Extracts a given range of bits from each input sample and presents it at the output.  The output type is ordinarily unsigned with binary point at zero, but can be Boolean when the slice is one bit wide.<P><P>Hardware notes: In hardware this block costs nothing."  block_version=10.1.3
  Threshold Select : Subsystem
  Det : Outport
  Timeout : Outport  Port=2
  tag scope: CS_LTS_Det_1=global, CS_LTS_Det_2=global
  wires:
  Gateway Out2/1 -> LTS Corr/3
  Gateway Out4/1 -> LTS Corr/2
  Logical1/1 -> LTS Corr Timeout/3(LTS 1), Concat/2, [CS_LTS_Det_1], Gateway Out8/1
  Inverter/1 -> Logical1/2
  Reset/1 -> S-R Latch/2(R), S-R Latch1/2(R), Gateway Out10/1
  Logical2/1 -> S-R Latch1/1(S), LTS Corr Timeout/4(LTS 2), Concat/1, [CS_LTS_Det_2], Gateway Out9/1
  S-R Latch/1(Q) -> Inverter/1, Logical2/3
  Gateway Out1/1 -> LTS Corr/4
  Gateway Out3/1 -> LTS Corr/5
  rx_I/1 -> Slice/1, Gateway Out4/1
  rx_Q/1 -> Slice1/1, Gateway Out2/1
  Correlator/1(R) -> Relational2/1, Gateway Out1/1
  Gateway Out7/1 -> LTS Corr/8
  Posedge3/1(Q) -> Logical1/1, Logical2/2, S-R Latch/1(S), Gateway Out3/1
  Logical3/1 -> Posedge3/1(D)
  Relational2/1 -> Logical3/2
  En/1 -> LTS Corr Timeout/1(Pkt Det), Logical3/1, Gateway Out6/1, Threshold Select/1(Pkt Det), Gateway Out5/1
  S-R Latch1/1(Q) -> Inverter1/1
  Inverter1/1 -> Logical2/1
  LTS Corr Timeout/1(Timeout) -> Timeout/1, Gateway Out7/1
  Slice/1 -> Correlator/1(Rx I)
  Slice1/1 -> Correlator/2(Rx Q)
  rx_IQ_valid/1 -> LTS Corr Timeout/2(IQ Valid), Correlator/3(rx_IQ_valid)
  Bus Creator/1 -> LTS Corr/6
  Bus Creator1/1 -> LTS Corr/7
  Gateway Out8/1 -> Bus Creator/1
  Gateway Out9/1 -> Bus Creator/2
  Gateway Out6/1 -> Bus Creator1/1
  Gateway Out10/1 -> Bus Creator1/2
  Threshold Select/1(Corr Thresh) -> Relational2/2
  RSSI/1 -> Threshold Select/2(RSSI)
  Concat/1 -> Det/1
  Gateway Out5/1 -> LTS Corr/1

== system wlan_phy_rx_pmd/Sync & Antenna Sel/PHY CCA ==
  LTS Sync : Inport
  LTS Timeout : Inport  Port=2
  IQ Valid : Inport  Port=3
  Det OFDM : Inport  Port=4
  Avg RSSI Vec : Inport  Port=5
  Reset OFDM : Inport  Port=6
  Det DSSS : Inport  Port=7
  Reset DSSS : Inport  Port=8
  Bus Selector1 : BusSelector  OutputSignals=RFA_RSSI,RFB_RSSI,RFC_RSSI,RFD_RSSI
  Gateway Out1 : xbsIndex_r4/Gateway Out  inherit_from_input=off  hdl_port=off  timing_constraint=None  locs_specified=off  LOCs={}  UseAsDAC=off  DACChannel='1'  block_type=gatewayout
  Gateway Out2 : xbsIndex_r4/Gateway Out  inherit_from_input=off  hdl_port=off  timing_constraint=None  locs_specified=off  LOCs={}  UseAsDAC=off  DACChannel='1'  block_type=gatewayout
  Gateway Out4 : xbsIndex_r4/Gateway Out  inherit_from_input=off  hdl_port=off  timing_constraint=None  locs_specified=off  LOCs={}  UseAsDAC=off  DACChannel='1'  block_type=gatewayout
  Logical1 : xbsIndex_r4/Logical  logical_function=OR  inputs=2  en=off  latency=0  precision=Full  arith_type=Unsigned  n_bits=16  bin_pt=0  align_bp=on  dbl_ovrd=off  block_type=logical
  Logical2 : xbsIndex_r4/Logical  logical_function=OR  inputs=4  en=off  latency=0  precision=Full  arith_type=Unsigned  n_bits=16  bin_pt=0  align_bp=on  dbl_ovrd=off  block_type=logical
  Logical3 : xbsIndex_r4/Logical  logical_function=AND  inputs=2  en=off  latency=0  precision=Full  arith_type=Unsigned  n_bits=16  bin_pt=0  align_bp=on  dbl_ovrd=off  block_type=logical
  Logical4 : xbsIndex_r4/Logical  logical_function=AND  inputs=2  en=off  latency=0  precision=Full  arith_type=Unsigned  n_bits=16  bin_pt=0  align_bp=on  dbl_ovrd=off  block_type=logical
  Logical5 : xbsIndex_r4/Logical  logical_function=OR  inputs=2  en=off  latency=0  precision=Full  arith_type=Unsigned  n_bits=16  bin_pt=0  align_bp=on  dbl_ovrd=off  block_type=logical
  PHY CCA : Scope  NumInputPorts=7
      settings: TimeRange=10000  YMin=-1~-1~0.95~-70~0~0~-0.25  YMax=1~1~1.05~80~1~60000~0.3  SaveName=ScopeData31
  PHY CCA Logic : Subsystem
  Rate-Length BUSY : Subsystem
  Relational{,1..3} (4 alike) : xbsIndex_r4/Relational  mode=a>b  en=off  latency=0  dbl_ovrd=off  block_type=relational
  S-R Latch1 : Subsystem
  S-R Latch2 : Subsystem
  S-R Latch3 : Subsystem
  Slice2 : xbsIndex_r4/Slice  nbits=1  boolean_output=on  mode=Upper Bit Location + Width  bit1=0  base1=MSB of Input  bit0=0  base0=LSB of Input  dbl_ovrd=off  block_type=slice
  tag scope: RX_CCA_BUSY=global, RX_END_OFDM_LAST_SAMP=global, RX_PHY_RATE_LENGTH_ACTIVE=global
  wires:
  [regRx_RSSI_CS_THRESH] -> Relational/2, Relational1/2, Relational2/2, Relational3/2
  Bus Selector1/1 -> Relational/1  "<RFA_RSSI>"
  Logical2/1 -> [RX_CCA_BUSY], Gateway Out2/1  "CCA_BUSY"
  Det OFDM/1 -> S-R Latch2/1(S)
  Logical1/1 -> S-R Latch2/2(R)
  Reset OFDM/1 -> Logical1/1
  LTS Timeout/1 -> Logical1/2
  S-R Latch2/1(Q) -> Logical3/2, Logical2/3
  IQ Valid/1 -> Rate-Length BUSY/1(IQ Valid)
  Logical3/1 -> Gateway Out4/1, Rate-Length BUSY/2(Start)
  LTS Sync/1 -> Slice2/1
  Avg RSSI Vec/1 -> Bus Selector1/1
  Slice2/1 -> Logical3/1
  Relational/1 -> PHY CCA Logic/1(Det A)
  Relational1/1 -> PHY CCA Logic/2(Det B)
  Bus Selector1/2 -> Relational1/1  "<RFB_RSSI>"
  PHY CCA Logic/1(BUSY) -> Logical2/2
  Bus Selector1/3 -> Relational2/1  "<RFC_RSSI>"
  Bus Selector1/4 -> Relational3/1  "<RFD_RSSI>"
  Relational2/1 -> PHY CCA Logic/3(Det C)
  Relational3/1 -> PHY CCA Logic/4(Det D)
  Gateway Out4/1 -> PHY CCA/1  "Start"
  Gateway Out1/1 -> PHY CCA/2  "RL Active"
  Gateway Out2/1 -> PHY CCA/3  "CCA Busy"
  [OFDM_RX_SIGNAL_VALID] -> S-R Latch1/1(S)
  S-R Latch1/1(Q) -> Logical2/1, [RX_PHY_RATE_LENGTH_ACTIVE], Gateway Out1/1
  Rate-Length BUSY/1(Pkt Done) -> [RX_END_OFDM_LAST_SAMP], Logical5/1
  Det DSSS/1 -> S-R Latch3/1(S)
  Reset DSSS/1 -> S-R Latch3/2(R)
  [regRx_DSSS_asserts_CCA_busy] -> Logical4/1
  S-R Latch3/1(Q) -> Logical4/2
  Logical4/1 -> Logical2/4
  Logical5/1 -> S-R Latch1/2(R)
  [RX_GLOBAL_RESET] -> Logical5/2

== system wlan_phy_rx_pmd/Sync & Antenna Sel/Pkt Det ==
  Reset OFDM : Inport
  Reset DSSS : Inport  Port=2
  Rx I Vec : Inport  Port=3
  Rx Q Vec : Inport  Port=4
  IQ Valid : Inport  Port=5
  RSSI Vec : Inport  Port=6
  Bus Selector : BusSelector  OutputSignals=RFA_I,RFB_I,RFC_I,RFD_I
  Bus Selector1 : BusSelector  OutputSignals=RFA_Q,RFB_Q,RFC_Q,RFD_Q
  Bus Selector2 : BusSelector  OutputSignals=RFA_RSSI,RFB_RSSI,RFC_RSSI,RFD_RSSI
  Bus Selector3 : BusSelector  OutputSignals=signal1,signal2,signal3,signal4
  Constant2 : xbsIndex_r4/Constant  const=0  gui_display_data_type=Boolean  arith_type=Boolean  n_bits=1  bin_pt=0  preci_type=Single  exp_width=8  frac_width=24  explicit_period=off  period=1  equ=P=C  opselect=C  inp2=PCIN>>17  opr=+  inp1=P  carry=CIN  dbl_ovrd=off  block_type=constant
      settings: block_version=VER_STRING_GOES_HERE
  Constant3 : xbsIndex_r4/Constant  const=0  gui_display_data_type=Boolean  arith_type=Boolean  n_bits=1  bin_pt=0  preci_type=Single  exp_width=8  frac_width=24  explicit_period=off  period=1  equ=P=C  opselect=C  inp2=PCIN>>17  opr=+  inp1=P  carry=CIN  dbl_ovrd=off  block_type=constant
      settings: block_version=VER_STRING_GOES_HERE
  DSSS Ant Sel Logic : Subsystem
  Ext Pkt Det : Subsystem
  Logical1 : xbsIndex_r4/Logical  logical_function=OR  inputs=2  en=off  latency=0  precision=Full  arith_type=Unsigned  n_bits=16  bin_pt=0  align_bp=on  dbl_ovrd=off  block_type=logical
  OFDM Ant Sel Logic : Subsystem
  RF A Det : Subsystem
  RF B Det : Subsystem
  Register{1..4} (4 alike) : xbsIndex_r4/Register  init=0  rst=off  en=off  dbl_ovrd=off  block_type=register
  Det OFDM : Outport
  Det DSSS : Outport  Port=2
  tag scope: regRx_pktDet_requireBothA=global, regRx_pktDet_requireBothB=global
  wires:
  Rx Q Vec/1 -> Bus Selector1/1
  IQ Valid/1 -> Bus Selector3/1
  RSSI Vec/1 -> Bus Selector2/1
  RF A Det/1(Det OFDM) -> OFDM Ant Sel Logic/2(Det A)
  OFDM Ant Sel Logic/1(Pkt Det) -> Logical1/2
  Rx I Vec/1 -> Bus Selector/1
  Logical1/1 -> Det OFDM/1
  Ext Pkt Det/1(Det) -> Logical1/1, OFDM Ant Sel Logic/1(Ext Det)
  Bus Selector/1 -> [RFA_I]  "<RFA_I>"
  Bus Selector/2 -> [RFB_I]  "<RFB_I>"
  Bus Selector/3 -> [RFC_I]  "<RFC_I>"
  Bus Selector/4 -> [RFD_I]  "<RFD_I>"
  Bus Selector1/1 -> [RFA_Q]  "<RFA_Q>"
  Bus Selector1/2 -> [RFB_Q]  "<RFB_Q>"
  Bus Selector1/3 -> [RFC_Q]  "<RFC_Q>"
  Bus Selector1/4 -> [RFD_Q]  "<RFD_Q>"
  Bus Selector3/1 -> [RFA_IQ_V]  "<signal1>"
  Bus Selector3/2 -> [RFB_IQ_V]  "<signal2>"
  Bus Selector3/3 -> [RFC_IQ_V]  "<signal3>"
  Bus Selector3/4 -> [RFD_IQ_V]  "<signal4>"
  Bus Selector2/1 -> [RFA_RSSI]  "<RFA_RSSI>"
  Bus Selector2/2 -> [RFB_RSSI]  "<RFB_RSSI>"
  Bus Selector2/3 -> [RFC_RSSI]  "<RFC_RSSI>"
  Bus Selector2/4 -> [RFD_RSSI]  "<RFD_RSSI>"
  [RFA_I] -> RF A Det/3(Rx I)
  [RFA_Q] -> RF A Det/4(Rx Q)
  [RFA_IQ_V] -> RF A Det/5(IQ Valid)
  [RFA_RSSI] -> RF A Det/6(RSSI)
  [RFB_I] -> RF B Det/3(Rx I)
  [RFB_Q] -> RF B Det/4(Rx Q)
  [RFB_IQ_V] -> RF B Det/5(IQ Valid)
  [RFB_RSSI] -> RF B Det/6(RSSI)
  [RFC_I] -> ?
  [RFC_Q] -> ?
  [RFC_IQ_V] -> ?
  [RFC_RSSI] -> ?
  [RFD_I] -> ?
  [RFD_Q] -> ?
  [RFD_IQ_V] -> ?
  [RFD_RSSI] -> ?
  RF A Det/2(Det DSSS) -> DSSS Ant Sel Logic/1(Det A)
  DSSS Ant Sel Logic/1(Pkt Det) -> Det DSSS/1
  Reset OFDM/1 -> Ext Pkt Det/1(Reset), RF A Det/1(Reset OFDM), RF B Det/1(Reset OFDM)
  Reset DSSS/1 -> RF A Det/2(Reset DSSS), RF B Det/2(Reset DSSS)
  Constant2/1 -> OFDM Ant Sel Logic/4(Det C), DSSS Ant Sel Logic/3(Det C)
  Constant3/1 -> OFDM Ant Sel Logic/5(Det D), DSSS Ant Sel Logic/4(Det D)
  RF B Det/1(Det OFDM) -> OFDM Ant Sel Logic/3(Det B)
  RF B Det/2(Det DSSS) -> DSSS Ant Sel Logic/2(Det B)
  Register3/1 -> Register1/1
  [regRx_pktDet_requireBoth] -> Register3/1, Register2/1
  Register2/1 -> Register4/1
  Register1/1 -> [regRx_pktDet_requireBothA]
  Register4/1 -> [regRx_pktDet_requireBothB]

== system wlan_phy_rx_pmd/Sync & Antenna Sel/Pkt Det AGC Ctrl ==
  note: Pkt det output to start/reset AGC
  Det OFDM : Inport
  Det DSSS : Inport  Port=2
  AGC Ctrl : Scope  NumInputPorts=4
      settings: YMin=-5~-5~-5~-5  YMax=5~5~5~5  SaveName=ScopeData24
  Gateway Out : xbsIndex_r4/Gateway Out  inherit_from_input=off  hdl_port=off  timing_constraint=None  locs_specified=off  LOCs={}  UseAsDAC=off  DACChannel='1'  block_type=gatewayout
  Gateway Out1 : xbsIndex_r4/Gateway Out  inherit_from_input=off  hdl_port=off  timing_constraint=None  locs_specified=off  LOCs={}  UseAsDAC=off  DACChannel='1'  block_type=gatewayout
  Logical : xbsIndex_r4/Logical  logical_function=OR  inputs=2  en=off  latency=0  precision=Full  arith_type=Unsigned  n_bits=16  bin_pt=0  align_bp=on  dbl_ovrd=off  block_type=logical
  Register1 : xbsIndex_r4/Register  init=0  rst=off  en=off  dbl_ovrd=off  block_type=register
  pkt_det_o : xbsIndex_r4/Gateway Out  inherit_from_input=off  hdl_port=on  timing_constraint=None  locs_specified=off  LOCs={}  UseAsDAC=off  DACChannel='1'  block_type=gatewayout
  wires:
  Register1/1 -> pkt_det_o/1
  Logical/1 -> Register1/1
  Det OFDM/1 -> Logical/1, Gateway Out/1
  Gateway Out/1 -> AGC Ctrl/1
  Gateway Out1/1 -> AGC Ctrl/2
  Det DSSS/1 -> Logical/2, Gateway Out1/1

== system wlan_phy_rx_pmd/Sync & Antenna Sel/Resets ==
  note: Handle rare case of a valid SIGNAL being received, sending
      | RX_START to MAC, then getting reset before the pkt is processed.
      | RX_START must be followed by RX_END for sane MAC state.
  OFDM Reset : Inport
  "DSSS Reset " : Inport  Port=2
  LTS Timeout : Inport  Port=3
  Constant1 : Constant  Value=0
  Inverter4 : xbsIndex_r4/Inverter  en=off  latency=0  dbl_ovrd=off  block_type=inv
  Logical1 : xbsIndex_r4/Logical  logical_function=AND  inputs=2  en=off  latency=0  precision=Full  arith_type=Unsigned  n_bits=16  bin_pt=0  align_bp=on  dbl_ovrd=off  block_type=logical
  Logical2 : xbsIndex_r4/Logical  logical_function=OR  inputs=4  en=off  latency=0  precision=Full  arith_type=Unsigned  n_bits=16  bin_pt=0  align_bp=on  dbl_ovrd=off  block_type=logical
  Logical3 : xbsIndex_r4/Logical  logical_function=OR  inputs=2  en=off  latency=0  precision=Full  arith_type=Unsigned  n_bits=16  bin_pt=0  align_bp=on  dbl_ovrd=off  block_type=logical
  Logical4 : xbsIndex_r4/Logical  logical_function=OR  inputs=3  en=off  latency=0  precision=Full  arith_type=Unsigned  n_bits=16  bin_pt=0  align_bp=on  dbl_ovrd=off  block_type=logical
  Logical5 : xbsIndex_r4/Logical  logical_function=OR  inputs=2  en=off  latency=0  precision=Full  arith_type=Unsigned  n_bits=16  bin_pt=0  align_bp=on  dbl_ovrd=off  block_type=logical
  Logical6 : xbsIndex_r4/Logical  logical_function=AND  inputs=2  en=off  latency=0  precision=Full  arith_type=Unsigned  n_bits=16  bin_pt=0  align_bp=on  dbl_ovrd=off  block_type=logical
  Mux : xbsIndex_r4/Mux  inputs=2  en=off  latency=0  precision=Full  arith_type=Unsigned  n_bits=16  bin_pt=14  quantization=Truncate  overflow=Wrap  dbl_ovrd=off  block_type=mux
  Negedge : Subsystem
  PHY_RX_RESET : xbsIndex_r4/Gateway In  gui_display_data_type=Boolean  arith_type=Boolean  n_bits=16  bin_pt=14  preci_type=Single  exp_width=8  frac_width=24  quantization="Round  (unbiased: +/- Inf)"  overflow=Saturate  period=1  dbl_ovrd=off  timing_constraint=None  locs_specified=off  LOCs={}  UseAsADC=off  ADCChannel='1'  inherit_from_input=off  hdl_port=on  block_type=gatewayin
  Register3 : xbsIndex_r4/Register  init=0  rst=off  en=off  dbl_ovrd=off  block_type=register
  Reset Gen : Subsystem
  Reset Gen1 : Subsystem
  " OFDM Reset" : Outport
  DSSS Reset : Outport  Port=2
  tag scope: RX_RESET_WHILE_ACTIVE=global
  wires:
  Logical1/1 -> [RX_RESET_WHILE_ACTIVE]
  Logical3/1 -> Logical1/1
  [RX_STARTED_TO_MAC] -> Logical1/2
  Register3/1 -> Logical2/4, Logical4/3, Logical3/1
  PHY_RX_RESET/1 -> Register3/1
  [RX_GLOBAL_RESET] -> Logical3/2, Logical2/3, Logical4/2
  Logical2/1 -> Reset Gen/1(Done)
  Reset Gen/1(Reset) -> " OFDM Reset"/1
  Constant1/1 -> PHY_RX_RESET/1
  LTS Timeout/1 -> Logical2/2
  Reset Gen1/1(Reset) -> DSSS Reset/1
  Logical4/1 -> Reset Gen1/1(Done)
  "DSSS Reset "/1 -> Logical4/1
  [RX_PHY_RATE_LENGTH_ACTIVE] -> Inverter4/1, Negedge/1(D)
  Inverter4/1 -> Logical6/2
  Logical6/1 -> Logical5/1
  Negedge/1(Q) -> Logical5/2
  OFDM Reset/1 -> Logical6/1, Mux/2
  Logical5/1 -> Mux/3
  Mux/1 -> Logical2/1
  [regRx_rateLength_holds_pktDet] -> Mux/1

== system wlan_phy_rx_pmd/CFO & Samp Buffer/CFO Correction/Sim Reset ==
  Constant4 : xbsIndex_r4/Constant  const=0  gui_display_data_type=Boolean  arith_type=Boolean  n_bits=16  bin_pt=14  preci_type=Single  exp_width=8  frac_width=24  explicit_period=on  period=1  equ=P=C  opselect=C  inp2=PCIN>>17  opr=+  inp1=P  carry=CIN  dbl_ovrd=off  block_type=constant
  Simulation Multiplexer : xbsIndex_r4/Simulation Multiplexer  sim_sel=1  hw_sel=2  block_type=simmux
      settings: infoedit="Distinguish input subsystems as \"simulation only\" and \"generation only\".  The input specified For Simulation will be used during Simulink simulation.  The input specified For Generation will be used during code generation.  This block will typically be used on the output of a HW Co-Simulation, Black Box, or ModelSim subsystem.<br><br>Hardware Notes: This block costs nothing."
  Subsystem : Subsystem
  Out1 : Outport
  wires:
  Subsystem/1(Out1) -> Simulation Multiplexer/1
  Constant4/1 -> Simulation Multiplexer/2
  Simulation Multiplexer/1 -> Out1/1

== system wlan_phy_rx_pmd/CFO & Samp Buffer/CFO Estimation/Conj Mult ==
  Valid : Inport
  A I : Inport  Port=2
  A Q : Inport  Port=3
  B I : Inport  Port=4
  B Q : Inport  Port=5
  AddSub : xbsIndex_r4/AddSub  mode=Addition  use_carryin=off  use_carryout=off  en=off  latency=1  precision=User Defined  arith_type="Signed  (2's comp)"  n_bits=17  bin_pt=14  quantization=Truncate  overflow=Flag as error  dbl_ovrd=off  use_behavioral_HDL=off  hw_selection=Fabric  pipelined=off  block_type=addsub
  AddSub1 : xbsIndex_r4/AddSub  mode=Subtraction  use_carryin=off  use_carryout=off  en=off  latency=1  precision=User Defined  arith_type="Signed  (2's comp)"  n_bits=17  bin_pt=14  quantization=Truncate  overflow=Flag as error  dbl_ovrd=off  use_behavioral_HDL=off  hw_selection=Fabric  pipelined=off  block_type=addsub
  Delay : xbsIndex_r4/Delay  rst=off  infoeditControl=Selection of Reset will increase slice count due to use of real FFs and instead of SRLs  en=off  latency=3  dbl_ovrd=off  reg_retiming=off  block_type=delay
  Mult{,1..3} (4 alike) : xbsIndex_r4/Mult  precision=User Defined  arith_type="Signed  (2's comp)"  n_bits=16  bin_pt=14  quantization=Truncate  overflow=Flag as error  en=off  latency=1  dbl_ovrd=off  use_behavioral_HDL=off  opt=Speed  use_embedded=on  optimum_pipeline=off  pipeline=on  use_rpm=on  placement_style=Triangular  block_type=mult
  Register{1..4} (4 alike) : xbsIndex_r4/Register  init=0  rst=off  en=on  dbl_ovrd=off  block_type=register
  " Valid" : Outport
  " I" : Outport  Port=2
  " Q" : Outport  Port=3
  wires:
  B Q/1 -> Register4/1
  Valid/1 -> Delay/1, Register1/2, Register2/2, Register3/2, Register4/2
  A I/1 -> Register1/1
  B I/1 -> Register2/1
  Mult/1 -> AddSub/1
  Mult1/1 -> AddSub/2
  AddSub/1 -> " I"/1
  Delay/1 -> " Valid"/1
  AddSub1/1 -> " Q"/1
  Register1/1 -> Mult/1, Mult2/1
  Register2/1 -> Mult/2, Mult3/1
  Register3/1 -> Mult1/1, Mult3/2
  Register4/1 -> Mult1/2, Mult2/2
  A Q/1 -> Register3/1
  Mult2/1 -> AddSub1/1
  Mult3/1 -> AddSub1/2
